simulink model "V2x_TC_TrafficLights_cs"  (Simulink 7.6)
settings: Solver=ode5  SolverMode=SingleTasking  StartTime=0.0  StopTime=38  FixedStep=1/100  RelTol=1e-3
PreLoadFcn:
  | check_NUTS_defined
  | 
  | assignin('base',['model_closed_' bdroot],false);addpath('../includes_V2x');
InitFcn:
  | PreScanExperimentInitFcn(bdroot)
StopFcn:
  | PreScanExperimentStopFcn(bdroot);
  | 
undefined symbols (not defined in the file or in its folder): acceleration, actorID, actorType, DiscIntNeverNeededParam, GPS_alt, GPS_lat, GPS_long, intmax, lightBarInUse, messageID, Newton, publicActorType, Raphson, rotZ, sigbuilder_block, sireneInUse, trafficLightPriority, turnAdvice, velocity, x_ini_VRML, y_ini_VRML, yawRate, z_ini_VRML
libraries: simulink x172, PreScanSimulinkLibrary x20, PreScanUsefulBlocks x11, simulink_extras x9, simulink_need_slupdate x1
(part 22 of 27)

== system V2x_TC_TrafficLights_cs/TrafficLightRoadSideNL_8/Infrastructure CAM generator/set CAM signals ==
  CAM generation signal : Inport
  warning : Inport  Port=2
  messageID : Inport  Port=3
  actorID : Inport  Port=4
  actorType : Inport  Port=5
  trafficLightPriority : Inport  Port=6
  GPS_lat : Inport  Port=7
  GPS_long : Inport  Port=8
  GPS_alt : Inport  Port=9
  rotZ : Inport  Port=10
  Mux : Mux  Inputs=9
  Switch : Switch  Criteria=u2 > Threshold  Threshold=0.5
  Unit Delay : UnitDelay  SampleTime=-1
  CAM_signals : Outport
  wires:
  rotZ/1 -> Mux/9
  GPS_alt/1 -> Mux/8
  GPS_long/1 -> Mux/7
  GPS_lat/1 -> Mux/6
  trafficLightPriority/1 -> Mux/5
  actorType/1 -> Mux/4
  actorID/1 -> Mux/3
  messageID/1 -> Mux/2
  warning/1 -> Mux/1
  Unit Delay/1 -> Switch/3
  Switch/1 -> CAM_signals/1, Unit Delay/1
  Mux/1 -> Switch/1
  CAM generation signal/1 -> Switch/2

== system V2x_TC_TrafficLights_cs/TrafficSignPoleNL_1/Infrastructure CAM generator/CAM generation ==
  Enable : EnablePort  StatesWhenEnabling=reset  PropagateVarSize=During execution
  Add : Sum  Inputs=+-
  Data Store Memory : DataStoreMemory  DataStoreName=CAM_time
  Data Store Read : DataStoreRead  DataStoreName=CAM_time  SampleTime=0.01
  Digital Clock : DigitalClock  SampleTime=0.01
  Merge : Merge
  if : If  IfExpression=u1 >= 1
  no : Subsystem  TreatAsAtomicUnit=on
  yes : Subsystem  TreatAsAtomicUnit=on
  CAN generation signal : Outport  OutputWhenDisabled=reset  InitialOutput=0
  wires:
  if/2 -> no/Action
  Merge/1 -> CAN generation signal/1
  no/1(Out1) -> Merge/2
  yes/1(Out1) -> Merge/1
  if/1 -> yes/Action
  Add/1 -> if/1
  Data Store Read/1 -> Add/2
  Digital Clock/1 -> Add/1

== system V2x_TC_TrafficLights_cs/TrafficSignPoleNL_1/Infrastructure CAM generator/Enabled Subsystem ==
  CAM_sig : Inport
  Enable : EnablePort  PropagateVarSize=During execution
  CAM_signals : Outport  OutputWhenDisabled=reset  InitialOutput=0
  wires:
  CAM_sig/1 -> CAM_signals/1

== system V2x_TC_TrafficLights_cs/TrafficSignPoleNL_1/Infrastructure CAM generator/coder ==
  R : Inport
  O : Inport  Port=2
  G : Inport  Port=3
  Add : Sum  Inputs=+++
  Gain : Gain  Gain=2
  Gain1 : Gain  Gain=3
  trafficLightPriority : Outport
  wires:
  Gain/1 -> Add/2
  Gain1/1 -> Add/3
  R/1 -> Add/1
  O/1 -> Gain/1
  Add/1 -> trafficLightPriority/1
  G/1 -> Gain1/1

== system V2x_TC_TrafficLights_cs/TrafficSignPoleNL_1/Infrastructure CAM generator/impulse generator ==
  CAM_time_step : Inport
  Add : Sum
  Compare To Zero : simulink/Logic and Bit Operations/Compare To Zero  SystemSampleTime=-1  relop=>  LogicOutDataTypeMode=boolean  ZeroCross=off
  Data Type Conversion : DataTypeConversion  OutDataTypeStr=boolean
  Detect Change : simulink/Logic and Bit Operations/Detect Change  SystemSampleTime=-1  vinit=0
  Digital Clock1 : DigitalClock  SampleTime=0.01
  Divide : Product  Inputs=*/
  Gain : Gain  Gain=pi
  Product : Product  RndMeth=Zero
  Trigonometric Function : Trigonometry
  constant : Constant
  experiment time step : Constant  Value=0.01  SampleTime=0
  enable : Outport
  wires:
  CAM_time_step/1 -> Divide/2
  Data Type Conversion/1 -> enable/1
  experiment time step/1 -> Add/2
  Add/1 -> Trigonometric Function/1
  Divide/1 -> Gain/1
  constant/1 -> Divide/1
  Product/1 -> Add/1
  Detect Change/1 -> Data Type Conversion/1
  Compare To Zero/1 -> Detect Change/1
  Trigonometric Function/1 -> Compare To Zero/1
  Gain/1 -> Product/2
  Digital Clock1/1 -> Product/1

== system V2x_TC_TrafficLights_cs/TrafficSignPoleNL_1/Infrastructure CAM generator/impulse generator1 ==
  CAM_time_step : Inport
  Add : Sum
  Compare To Zero : simulink/Logic and Bit Operations/Compare To Zero  SystemSampleTime=-1  relop=>  LogicOutDataTypeMode=boolean  ZeroCross=off
  Data Type Conversion : DataTypeConversion  OutDataTypeStr=boolean
  Detect Change : simulink/Logic and Bit Operations/Detect Change  SystemSampleTime=-1  vinit=0
  Digital Clock1 : DigitalClock  SampleTime=0.01
  Divide : Product  Inputs=*/
  Gain : Gain  Gain=pi
  Product : Product  RndMeth=Zero
  Trigonometric Function : Trigonometry
  constant : Constant
  experiment time step : Constant  Value=0.01  SampleTime=0
  enable : Outport
  wires:
  Digital Clock1/1 -> Product/1
  Gain/1 -> Product/2
  Trigonometric Function/1 -> Compare To Zero/1
  Compare To Zero/1 -> Detect Change/1
  Detect Change/1 -> Data Type Conversion/1
  Product/1 -> Add/1
  constant/1 -> Divide/1
  Divide/1 -> Gain/1
  Add/1 -> Trigonometric Function/1
  experiment time step/1 -> Add/2
  Data Type Conversion/1 -> enable/1
  CAM_time_step/1 -> Divide/2

== system V2x_TC_TrafficLights_cs/TrafficSignPoleNL_1/Infrastructure CAM generator/set CAM signals ==
  CAM generation signal : Inport
  warning : Inport  Port=2
  messageID : Inport  Port=3
  actorID : Inport  Port=4
  actorType : Inport  Port=5
  trafficLightPriority : Inport  Port=6
  GPS_lat : Inport  Port=7
  GPS_long : Inport  Port=8
  GPS_alt : Inport  Port=9
  rotZ : Inport  Port=10
  Mux : Mux  Inputs=9
  Switch : Switch  Criteria=u2 > Threshold  Threshold=0.5
  Unit Delay : UnitDelay  SampleTime=-1
  CAM_signals : Outport
  wires:
  rotZ/1 -> Mux/9
  GPS_alt/1 -> Mux/8
  GPS_long/1 -> Mux/7
  GPS_lat/1 -> Mux/6
  trafficLightPriority/1 -> Mux/5
  actorType/1 -> Mux/4
  actorID/1 -> Mux/3
  messageID/1 -> Mux/2
  warning/1 -> Mux/1
  Unit Delay/1 -> Switch/3
  Switch/1 -> CAM_signals/1, Unit Delay/1
  Mux/1 -> Switch/1
  CAM generation signal/1 -> Switch/2

== system V2x_TC_TrafficLights_cs/TrafficSignPoleNL_10/Infrastructure CAM generator/CAM generation ==
  Enable : EnablePort  StatesWhenEnabling=reset  PropagateVarSize=During execution
  Add : Sum  Inputs=+-
  Data Store Memory : DataStoreMemory  DataStoreName=CAM_time
  Data Store Read : DataStoreRead  DataStoreName=CAM_time  SampleTime=0.01
  Digital Clock : DigitalClock  SampleTime=0.01
  Merge : Merge
  if : If  IfExpression=u1 >= 1
  no : Subsystem  TreatAsAtomicUnit=on
  yes : Subsystem  TreatAsAtomicUnit=on
  CAN generation signal : Outport  OutputWhenDisabled=reset  InitialOutput=0
  wires:
  if/2 -> no/Action
  Merge/1 -> CAN generation signal/1
  no/1(Out1) -> Merge/2
  yes/1(Out1) -> Merge/1
  if/1 -> yes/Action
  Add/1 -> if/1
  Data Store Read/1 -> Add/2
  Digital Clock/1 -> Add/1

== system V2x_TC_TrafficLights_cs/TrafficSignPoleNL_10/Infrastructure CAM generator/Enabled Subsystem ==
  CAM_sig : Inport
  Enable : EnablePort  PropagateVarSize=During execution
  CAM_signals : Outport  OutputWhenDisabled=reset  InitialOutput=0
  wires:
  CAM_sig/1 -> CAM_signals/1

== system V2x_TC_TrafficLights_cs/TrafficSignPoleNL_10/Infrastructure CAM generator/coder ==
  R : Inport
  O : Inport  Port=2
  G : Inport  Port=3
  Add : Sum  Inputs=+++
  Gain : Gain  Gain=2
  Gain1 : Gain  Gain=3
  trafficLightPriority : Outport
  wires:
  Gain/1 -> Add/2
  Gain1/1 -> Add/3
  R/1 -> Add/1
  O/1 -> Gain/1
  Add/1 -> trafficLightPriority/1
  G/1 -> Gain1/1

== system V2x_TC_TrafficLights_cs/TrafficSignPoleNL_10/Infrastructure CAM generator/impulse generator ==
  CAM_time_step : Inport
  Add : Sum
  Compare To Zero : simulink/Logic and Bit Operations/Compare To Zero  SystemSampleTime=-1  relop=>  LogicOutDataTypeMode=boolean  ZeroCross=off
  Data Type Conversion : DataTypeConversion  OutDataTypeStr=boolean
  Detect Change : simulink/Logic and Bit Operations/Detect Change  SystemSampleTime=-1  vinit=0
  Digital Clock1 : DigitalClock  SampleTime=0.01
  Divide : Product  Inputs=*/
  Gain : Gain  Gain=pi
  Product : Product  RndMeth=Zero
  Trigonometric Function : Trigonometry
  constant : Constant
  experiment time step : Constant  Value=0.01  SampleTime=0
  enable : Outport
  wires:
  CAM_time_step/1 -> Divide/2
  Data Type Conversion/1 -> enable/1
  experiment time step/1 -> Add/2
  Add/1 -> Trigonometric Function/1
  Divide/1 -> Gain/1
  constant/1 -> Divide/1
  Product/1 -> Add/1
  Detect Change/1 -> Data Type Conversion/1
  Compare To Zero/1 -> Detect Change/1
  Trigonometric Function/1 -> Compare To Zero/1
  Gain/1 -> Product/2
  Digital Clock1/1 -> Product/1

== system V2x_TC_TrafficLights_cs/TrafficSignPoleNL_10/Infrastructure CAM generator/impulse generator1 ==
  CAM_time_step : Inport
  Add : Sum
  Compare To Zero : simulink/Logic and Bit Operations/Compare To Zero  SystemSampleTime=-1  relop=>  LogicOutDataTypeMode=boolean  ZeroCross=off
  Data Type Conversion : DataTypeConversion  OutDataTypeStr=boolean
  Detect Change : simulink/Logic and Bit Operations/Detect Change  SystemSampleTime=-1  vinit=0
  Digital Clock1 : DigitalClock  SampleTime=0.01
  Divide : Product  Inputs=*/
  Gain : Gain  Gain=pi
  Product : Product  RndMeth=Zero
  Trigonometric Function : Trigonometry
  constant : Constant
  experiment time step : Constant  Value=0.01  SampleTime=0
  enable : Outport
  wires:
  Digital Clock1/1 -> Product/1
  Gain/1 -> Product/2
  Trigonometric Function/1 -> Compare To Zero/1
  Compare To Zero/1 -> Detect Change/1
  Detect Change/1 -> Data Type Conversion/1
  Product/1 -> Add/1
  constant/1 -> Divide/1
  Divide/1 -> Gain/1
  Add/1 -> Trigonometric Function/1
  experiment time step/1 -> Add/2
  Data Type Conversion/1 -> enable/1
  CAM_time_step/1 -> Divide/2

== system V2x_TC_TrafficLights_cs/TrafficSignPoleNL_10/Infrastructure CAM generator/set CAM signals ==
  CAM generation signal : Inport
  warning : Inport  Port=2
  messageID : Inport  Port=3
  actorID : Inport  Port=4
  actorType : Inport  Port=5
  trafficLightPriority : Inport  Port=6
  GPS_lat : Inport  Port=7
  GPS_long : Inport  Port=8
  GPS_alt : Inport  Port=9
  rotZ : Inport  Port=10
  Mux : Mux  Inputs=9
  Switch : Switch  Criteria=u2 > Threshold  Threshold=0.5
  Unit Delay : UnitDelay  SampleTime=-1
  CAM_signals : Outport
  wires:
  rotZ/1 -> Mux/9
  GPS_alt/1 -> Mux/8
  GPS_long/1 -> Mux/7
  GPS_lat/1 -> Mux/6
  trafficLightPriority/1 -> Mux/5
  actorType/1 -> Mux/4
  actorID/1 -> Mux/3
  messageID/1 -> Mux/2
  warning/1 -> Mux/1
  Unit Delay/1 -> Switch/3
  Switch/1 -> CAM_signals/1, Unit Delay/1
  Mux/1 -> Switch/1
  CAM generation signal/1 -> Switch/2

== system V2x_TC_TrafficLights_cs/TrafficSignPoleNL_2/Infrastructure CAM generator/CAM generation ==
  Enable : EnablePort  StatesWhenEnabling=reset  PropagateVarSize=During execution
  Add : Sum  Inputs=+-
  Data Store Memory : DataStoreMemory  DataStoreName=CAM_time
  Data Store Read : DataStoreRead  DataStoreName=CAM_time  SampleTime=0.01
  Digital Clock : DigitalClock  SampleTime=0.01
  Merge : Merge
  if : If  IfExpression=u1 >= 1
  no : Subsystem  TreatAsAtomicUnit=on
  yes : Subsystem  TreatAsAtomicUnit=on
  CAN generation signal : Outport  OutputWhenDisabled=reset  InitialOutput=0
  wires:
  if/2 -> no/Action
  Merge/1 -> CAN generation signal/1
  no/1(Out1) -> Merge/2
  yes/1(Out1) -> Merge/1
  if/1 -> yes/Action
  Add/1 -> if/1
  Data Store Read/1 -> Add/2
  Digital Clock/1 -> Add/1

== system V2x_TC_TrafficLights_cs/TrafficSignPoleNL_2/Infrastructure CAM generator/Enabled Subsystem ==
  CAM_sig : Inport
  Enable : EnablePort  PropagateVarSize=During execution
  CAM_signals : Outport  OutputWhenDisabled=reset  InitialOutput=0
  wires:
  CAM_sig/1 -> CAM_signals/1

== system V2x_TC_TrafficLights_cs/TrafficSignPoleNL_2/Infrastructure CAM generator/coder ==
  R : Inport
  O : Inport  Port=2
  G : Inport  Port=3
  Add : Sum  Inputs=+++
  Gain : Gain  Gain=2
  Gain1 : Gain  Gain=3
  trafficLightPriority : Outport
  wires:
  Gain/1 -> Add/2
  Gain1/1 -> Add/3
  R/1 -> Add/1
  O/1 -> Gain/1
  Add/1 -> trafficLightPriority/1
  G/1 -> Gain1/1

== system V2x_TC_TrafficLights_cs/TrafficSignPoleNL_2/Infrastructure CAM generator/impulse generator ==
  CAM_time_step : Inport
  Add : Sum
  Compare To Zero : simulink/Logic and Bit Operations/Compare To Zero  SystemSampleTime=-1  relop=>  LogicOutDataTypeMode=boolean  ZeroCross=off
  Data Type Conversion : DataTypeConversion  OutDataTypeStr=boolean
  Detect Change : simulink/Logic and Bit Operations/Detect Change  SystemSampleTime=-1  vinit=0
  Digital Clock1 : DigitalClock  SampleTime=0.01
  Divide : Product  Inputs=*/
  Gain : Gain  Gain=pi
  Product : Product  RndMeth=Zero
  Trigonometric Function : Trigonometry
  constant : Constant
  experiment time step : Constant  Value=0.01  SampleTime=0
  enable : Outport
  wires:
  CAM_time_step/1 -> Divide/2
  Data Type Conversion/1 -> enable/1
  experiment time step/1 -> Add/2
  Add/1 -> Trigonometric Function/1
  Divide/1 -> Gain/1
  constant/1 -> Divide/1
  Product/1 -> Add/1
  Detect Change/1 -> Data Type Conversion/1
  Compare To Zero/1 -> Detect Change/1
  Trigonometric Function/1 -> Compare To Zero/1
  Gain/1 -> Product/2
  Digital Clock1/1 -> Product/1

== system V2x_TC_TrafficLights_cs/TrafficSignPoleNL_2/Infrastructure CAM generator/impulse generator1 ==
  CAM_time_step : Inport
  Add : Sum
  Compare To Zero : simulink/Logic and Bit Operations/Compare To Zero  SystemSampleTime=-1  relop=>  LogicOutDataTypeMode=boolean  ZeroCross=off
  Data Type Conversion : DataTypeConversion  OutDataTypeStr=boolean
  Detect Change : simulink/Logic and Bit Operations/Detect Change  SystemSampleTime=-1  vinit=0
  Digital Clock1 : DigitalClock  SampleTime=0.01
  Divide : Product  Inputs=*/
  Gain : Gain  Gain=pi
  Product : Product  RndMeth=Zero
  Trigonometric Function : Trigonometry
  constant : Constant
  experiment time step : Constant  Value=0.01  SampleTime=0
  enable : Outport
  wires:
  Digital Clock1/1 -> Product/1
  Gain/1 -> Product/2
  Trigonometric Function/1 -> Compare To Zero/1
  Compare To Zero/1 -> Detect Change/1
  Detect Change/1 -> Data Type Conversion/1
  Product/1 -> Add/1
  constant/1 -> Divide/1
  Divide/1 -> Gain/1
  Add/1 -> Trigonometric Function/1
  experiment time step/1 -> Add/2
  Data Type Conversion/1 -> enable/1
  CAM_time_step/1 -> Divide/2

== system V2x_TC_TrafficLights_cs/TrafficSignPoleNL_2/Infrastructure CAM generator/set CAM signals ==
  CAM generation signal : Inport
  warning : Inport  Port=2
  messageID : Inport  Port=3
  actorID : Inport  Port=4
  actorType : Inport  Port=5
  trafficLightPriority : Inport  Port=6
  GPS_lat : Inport  Port=7
  GPS_long : Inport  Port=8
  GPS_alt : Inport  Port=9
  rotZ : Inport  Port=10
  Mux : Mux  Inputs=9
  Switch : Switch  Criteria=u2 > Threshold  Threshold=0.5
  Unit Delay : UnitDelay  SampleTime=-1
  CAM_signals : Outport
  wires:
  rotZ/1 -> Mux/9
  GPS_alt/1 -> Mux/8
  GPS_long/1 -> Mux/7
  GPS_lat/1 -> Mux/6
  trafficLightPriority/1 -> Mux/5
  actorType/1 -> Mux/4
  actorID/1 -> Mux/3
  messageID/1 -> Mux/2
  warning/1 -> Mux/1
  Unit Delay/1 -> Switch/3
  Switch/1 -> CAM_signals/1, Unit Delay/1
  Mux/1 -> Switch/1
  CAM generation signal/1 -> Switch/2

== system V2x_TC_TrafficLights_cs/TrafficSignPoleNL_3/Infrastructure CAM generator/CAM generation ==
  Enable : EnablePort  StatesWhenEnabling=reset  PropagateVarSize=During execution
  Add : Sum  Inputs=+-
  Data Store Memory : DataStoreMemory  DataStoreName=CAM_time
  Data Store Read : DataStoreRead  DataStoreName=CAM_time  SampleTime=0.01
  Digital Clock : DigitalClock  SampleTime=0.01
  Merge : Merge
  if : If  IfExpression=u1 >= 1
  no : Subsystem  TreatAsAtomicUnit=on
  yes : Subsystem  TreatAsAtomicUnit=on
  CAN generation signal : Outport  OutputWhenDisabled=reset  InitialOutput=0
  wires:
  if/2 -> no/Action
  Merge/1 -> CAN generation signal/1
  no/1(Out1) -> Merge/2
  yes/1(Out1) -> Merge/1
  if/1 -> yes/Action
  Add/1 -> if/1
  Data Store Read/1 -> Add/2
  Digital Clock/1 -> Add/1

== system V2x_TC_TrafficLights_cs/TrafficSignPoleNL_3/Infrastructure CAM generator/Enabled Subsystem ==
  CAM_sig : Inport
  Enable : EnablePort  PropagateVarSize=During execution
  CAM_signals : Outport  OutputWhenDisabled=reset  InitialOutput=0
  wires:
  CAM_sig/1 -> CAM_signals/1

== system V2x_TC_TrafficLights_cs/TrafficSignPoleNL_3/Infrastructure CAM generator/coder ==
  R : Inport
  O : Inport  Port=2
  G : Inport  Port=3
  Add : Sum  Inputs=+++
  Gain : Gain  Gain=2
  Gain1 : Gain  Gain=3
  trafficLightPriority : Outport
  wires:
  Gain/1 -> Add/2
  Gain1/1 -> Add/3
  R/1 -> Add/1
  O/1 -> Gain/1
  Add/1 -> trafficLightPriority/1
  G/1 -> Gain1/1

== system V2x_TC_TrafficLights_cs/TrafficSignPoleNL_3/Infrastructure CAM generator/impulse generator ==
  CAM_time_step : Inport
  Add : Sum
  Compare To Zero : simulink/Logic and Bit Operations/Compare To Zero  SystemSampleTime=-1  relop=>  LogicOutDataTypeMode=boolean  ZeroCross=off
  Data Type Conversion : DataTypeConversion  OutDataTypeStr=boolean
  Detect Change : simulink/Logic and Bit Operations/Detect Change  SystemSampleTime=-1  vinit=0
  Digital Clock1 : DigitalClock  SampleTime=0.01
  Divide : Product  Inputs=*/
  Gain : Gain  Gain=pi
  Product : Product  RndMeth=Zero
  Trigonometric Function : Trigonometry
  constant : Constant
  experiment time step : Constant  Value=0.01  SampleTime=0
  enable : Outport
  wires:
  CAM_time_step/1 -> Divide/2
  Data Type Conversion/1 -> enable/1
  experiment time step/1 -> Add/2
  Add/1 -> Trigonometric Function/1
  Divide/1 -> Gain/1
  constant/1 -> Divide/1
  Product/1 -> Add/1
  Detect Change/1 -> Data Type Conversion/1
  Compare To Zero/1 -> Detect Change/1
  Trigonometric Function/1 -> Compare To Zero/1
  Gain/1 -> Product/2
  Digital Clock1/1 -> Product/1

== system V2x_TC_TrafficLights_cs/TrafficSignPoleNL_3/Infrastructure CAM generator/impulse generator1 ==
  CAM_time_step : Inport
  Add : Sum
  Compare To Zero : simulink/Logic and Bit Operations/Compare To Zero  SystemSampleTime=-1  relop=>  LogicOutDataTypeMode=boolean  ZeroCross=off
  Data Type Conversion : DataTypeConversion  OutDataTypeStr=boolean
  Detect Change : simulink/Logic and Bit Operations/Detect Change  SystemSampleTime=-1  vinit=0
  Digital Clock1 : DigitalClock  SampleTime=0.01
  Divide : Product  Inputs=*/
  Gain : Gain  Gain=pi
  Product : Product  RndMeth=Zero
  Trigonometric Function : Trigonometry
  constant : Constant
  experiment time step : Constant  Value=0.01  SampleTime=0
  enable : Outport
  wires:
  Digital Clock1/1 -> Product/1
  Gain/1 -> Product/2
  Trigonometric Function/1 -> Compare To Zero/1
  Compare To Zero/1 -> Detect Change/1
  Detect Change/1 -> Data Type Conversion/1
  Product/1 -> Add/1
  constant/1 -> Divide/1
  Divide/1 -> Gain/1
  Add/1 -> Trigonometric Function/1
  experiment time step/1 -> Add/2
  Data Type Conversion/1 -> enable/1
  CAM_time_step/1 -> Divide/2

== system V2x_TC_TrafficLights_cs/TrafficSignPoleNL_3/Infrastructure CAM generator/set CAM signals ==
  CAM generation signal : Inport
  warning : Inport  Port=2
  messageID : Inport  Port=3
  actorID : Inport  Port=4
  actorType : Inport  Port=5
  trafficLightPriority : Inport  Port=6
  GPS_lat : Inport  Port=7
  GPS_long : Inport  Port=8
  GPS_alt : Inport  Port=9
  rotZ : Inport  Port=10
  Mux : Mux  Inputs=9
  Switch : Switch  Criteria=u2 > Threshold  Threshold=0.5
  Unit Delay : UnitDelay  SampleTime=-1
  CAM_signals : Outport
  wires:
  rotZ/1 -> Mux/9
  GPS_alt/1 -> Mux/8
  GPS_long/1 -> Mux/7
  GPS_lat/1 -> Mux/6
  trafficLightPriority/1 -> Mux/5
  actorType/1 -> Mux/4
  actorID/1 -> Mux/3
  messageID/1 -> Mux/2
  warning/1 -> Mux/1
  Unit Delay/1 -> Switch/3
  Switch/1 -> CAM_signals/1, Unit Delay/1
  Mux/1 -> Switch/1
  CAM generation signal/1 -> Switch/2

== system V2x_TC_TrafficLights_cs/TrafficSignPoleNL_4/Infrastructure CAM generator/CAM generation ==
  Enable : EnablePort  StatesWhenEnabling=reset  PropagateVarSize=During execution
  Add : Sum  Inputs=+-
  Data Store Memory : DataStoreMemory  DataStoreName=CAM_time
  Data Store Read : DataStoreRead  DataStoreName=CAM_time  SampleTime=0.01
  Digital Clock : DigitalClock  SampleTime=0.01
  Merge : Merge
  if : If  IfExpression=u1 >= 1
  no : Subsystem  TreatAsAtomicUnit=on
  yes : Subsystem  TreatAsAtomicUnit=on
  CAN generation signal : Outport  OutputWhenDisabled=reset  InitialOutput=0
  wires:
  if/2 -> no/Action
  Merge/1 -> CAN generation signal/1
  no/1(Out1) -> Merge/2
  yes/1(Out1) -> Merge/1
  if/1 -> yes/Action
  Add/1 -> if/1
  Data Store Read/1 -> Add/2
  Digital Clock/1 -> Add/1

== system V2x_TC_TrafficLights_cs/TrafficSignPoleNL_4/Infrastructure CAM generator/Enabled Subsystem ==
  CAM_sig : Inport
  Enable : EnablePort  PropagateVarSize=During execution
  CAM_signals : Outport  OutputWhenDisabled=reset  InitialOutput=0
  wires:
  CAM_sig/1 -> CAM_signals/1

== system V2x_TC_TrafficLights_cs/TrafficSignPoleNL_4/Infrastructure CAM generator/coder ==
  R : Inport
  O : Inport  Port=2
  G : Inport  Port=3
  Add : Sum  Inputs=+++
  Gain : Gain  Gain=2
  Gain1 : Gain  Gain=3
  trafficLightPriority : Outport
  wires:
  Gain/1 -> Add/2
  Gain1/1 -> Add/3
  R/1 -> Add/1
  O/1 -> Gain/1
  Add/1 -> trafficLightPriority/1
  G/1 -> Gain1/1

== system V2x_TC_TrafficLights_cs/TrafficSignPoleNL_4/Infrastructure CAM generator/impulse generator ==
  CAM_time_step : Inport
  Add : Sum
  Compare To Zero : simulink/Logic and Bit Operations/Compare To Zero  SystemSampleTime=-1  relop=>  LogicOutDataTypeMode=boolean  ZeroCross=off
  Data Type Conversion : DataTypeConversion  OutDataTypeStr=boolean
  Detect Change : simulink/Logic and Bit Operations/Detect Change  SystemSampleTime=-1  vinit=0
  Digital Clock1 : DigitalClock  SampleTime=0.01
  Divide : Product  Inputs=*/
  Gain : Gain  Gain=pi
  Product : Product  RndMeth=Zero
  Trigonometric Function : Trigonometry
  constant : Constant
  experiment time step : Constant  Value=0.01  SampleTime=0
  enable : Outport
  wires:
  CAM_time_step/1 -> Divide/2
  Data Type Conversion/1 -> enable/1
  experiment time step/1 -> Add/2
  Add/1 -> Trigonometric Function/1
  Divide/1 -> Gain/1
  constant/1 -> Divide/1
  Product/1 -> Add/1
  Detect Change/1 -> Data Type Conversion/1
  Compare To Zero/1 -> Detect Change/1
  Trigonometric Function/1 -> Compare To Zero/1
  Gain/1 -> Product/2
  Digital Clock1/1 -> Product/1

== system V2x_TC_TrafficLights_cs/TrafficSignPoleNL_4/Infrastructure CAM generator/impulse generator1 ==
  CAM_time_step : Inport
  Add : Sum
  Compare To Zero : simulink/Logic and Bit Operations/Compare To Zero  SystemSampleTime=-1  relop=>  LogicOutDataTypeMode=boolean  ZeroCross=off
  Data Type Conversion : DataTypeConversion  OutDataTypeStr=boolean
  Detect Change : simulink/Logic and Bit Operations/Detect Change  SystemSampleTime=-1  vinit=0
  Digital Clock1 : DigitalClock  SampleTime=0.01
  Divide : Product  Inputs=*/
  Gain : Gain  Gain=pi
  Product : Product  RndMeth=Zero
  Trigonometric Function : Trigonometry
  constant : Constant
  experiment time step : Constant  Value=0.01  SampleTime=0
  enable : Outport
  wires:
  Digital Clock1/1 -> Product/1
  Gain/1 -> Product/2
  Trigonometric Function/1 -> Compare To Zero/1
  Compare To Zero/1 -> Detect Change/1
  Detect Change/1 -> Data Type Conversion/1
  Product/1 -> Add/1
  constant/1 -> Divide/1
  Divide/1 -> Gain/1
  Add/1 -> Trigonometric Function/1
  experiment time step/1 -> Add/2
  Data Type Conversion/1 -> enable/1
  CAM_time_step/1 -> Divide/2

== system V2x_TC_TrafficLights_cs/TrafficSignPoleNL_4/Infrastructure CAM generator/set CAM signals ==
  CAM generation signal : Inport
  warning : Inport  Port=2
  messageID : Inport  Port=3
  actorID : Inport  Port=4
  actorType : Inport  Port=5
  trafficLightPriority : Inport  Port=6
  GPS_lat : Inport  Port=7
  GPS_long : Inport  Port=8
  GPS_alt : Inport  Port=9
  rotZ : Inport  Port=10
  Mux : Mux  Inputs=9
  Switch : Switch  Criteria=u2 > Threshold  Threshold=0.5
  Unit Delay : UnitDelay  SampleTime=-1
  CAM_signals : Outport
  wires:
  rotZ/1 -> Mux/9
  GPS_alt/1 -> Mux/8
  GPS_long/1 -> Mux/7
  GPS_lat/1 -> Mux/6
  trafficLightPriority/1 -> Mux/5
  actorType/1 -> Mux/4
  actorID/1 -> Mux/3
  messageID/1 -> Mux/2
  warning/1 -> Mux/1
  Unit Delay/1 -> Switch/3
  Switch/1 -> CAM_signals/1, Unit Delay/1
  Mux/1 -> Switch/1
  CAM generation signal/1 -> Switch/2

== system V2x_TC_TrafficLights_cs/TrafficSignPoleNL_5/Infrastructure CAM generator/CAM generation ==
  Enable : EnablePort  StatesWhenEnabling=reset  PropagateVarSize=During execution
  Add : Sum  Inputs=+-
  Data Store Memory : DataStoreMemory  DataStoreName=CAM_time
  Data Store Read : DataStoreRead  DataStoreName=CAM_time  SampleTime=0.01
  Digital Clock : DigitalClock  SampleTime=0.01
  Merge : Merge
  if : If  IfExpression=u1 >= 1
  no : Subsystem  TreatAsAtomicUnit=on
  yes : Subsystem  TreatAsAtomicUnit=on
  CAN generation signal : Outport  OutputWhenDisabled=reset  InitialOutput=0
  wires:
  if/2 -> no/Action
  Merge/1 -> CAN generation signal/1
  no/1(Out1) -> Merge/2
  yes/1(Out1) -> Merge/1
  if/1 -> yes/Action
  Add/1 -> if/1
  Data Store Read/1 -> Add/2
  Digital Clock/1 -> Add/1

== system V2x_TC_TrafficLights_cs/TrafficSignPoleNL_5/Infrastructure CAM generator/Enabled Subsystem ==
  CAM_sig : Inport
  Enable : EnablePort  PropagateVarSize=During execution
  CAM_signals : Outport  OutputWhenDisabled=reset  InitialOutput=0
  wires:
  CAM_sig/1 -> CAM_signals/1

== system V2x_TC_TrafficLights_cs/TrafficSignPoleNL_5/Infrastructure CAM generator/coder ==
  R : Inport
  O : Inport  Port=2
  G : Inport  Port=3
  Add : Sum  Inputs=+++
  Gain : Gain  Gain=2
  Gain1 : Gain  Gain=3
  trafficLightPriority : Outport
  wires:
  Gain/1 -> Add/2
  Gain1/1 -> Add/3
  R/1 -> Add/1
  O/1 -> Gain/1
  Add/1 -> trafficLightPriority/1
  G/1 -> Gain1/1

== system V2x_TC_TrafficLights_cs/TrafficSignPoleNL_5/Infrastructure CAM generator/impulse generator ==
  CAM_time_step : Inport
  Add : Sum
  Compare To Zero : simulink/Logic and Bit Operations/Compare To Zero  SystemSampleTime=-1  relop=>  LogicOutDataTypeMode=boolean  ZeroCross=off
  Data Type Conversion : DataTypeConversion  OutDataTypeStr=boolean
  Detect Change : simulink/Logic and Bit Operations/Detect Change  SystemSampleTime=-1  vinit=0
  Digital Clock1 : DigitalClock  SampleTime=0.01
  Divide : Product  Inputs=*/
  Gain : Gain  Gain=pi
  Product : Product  RndMeth=Zero
  Trigonometric Function : Trigonometry
  constant : Constant
  experiment time step : Constant  Value=0.01  SampleTime=0
  enable : Outport
  wires:
  CAM_time_step/1 -> Divide/2
  Data Type Conversion/1 -> enable/1
  experiment time step/1 -> Add/2
  Add/1 -> Trigonometric Function/1
  Divide/1 -> Gain/1
  constant/1 -> Divide/1
  Product/1 -> Add/1
  Detect Change/1 -> Data Type Conversion/1
  Compare To Zero/1 -> Detect Change/1
  Trigonometric Function/1 -> Compare To Zero/1
  Gain/1 -> Product/2
  Digital Clock1/1 -> Product/1

== system V2x_TC_TrafficLights_cs/TrafficSignPoleNL_5/Infrastructure CAM generator/impulse generator1 ==
  CAM_time_step : Inport
  Add : Sum
  Compare To Zero : simulink/Logic and Bit Operations/Compare To Zero  SystemSampleTime=-1  relop=>  LogicOutDataTypeMode=boolean  ZeroCross=off
  Data Type Conversion : DataTypeConversion  OutDataTypeStr=boolean
  Detect Change : simulink/Logic and Bit Operations/Detect Change  SystemSampleTime=-1  vinit=0
  Digital Clock1 : DigitalClock  SampleTime=0.01
  Divide : Product  Inputs=*/
  Gain : Gain  Gain=pi
  Product : Product  RndMeth=Zero
  Trigonometric Function : Trigonometry
  constant : Constant
  experiment time step : Constant  Value=0.01  SampleTime=0
  enable : Outport
  wires:
  Digital Clock1/1 -> Product/1
  Gain/1 -> Product/2
  Trigonometric Function/1 -> Compare To Zero/1
  Compare To Zero/1 -> Detect Change/1
  Detect Change/1 -> Data Type Conversion/1
  Product/1 -> Add/1
  constant/1 -> Divide/1
  Divide/1 -> Gain/1
  Add/1 -> Trigonometric Function/1
  experiment time step/1 -> Add/2
  Data Type Conversion/1 -> enable/1
  CAM_time_step/1 -> Divide/2

== system V2x_TC_TrafficLights_cs/TrafficSignPoleNL_5/Infrastructure CAM generator/set CAM signals ==
  CAM generation signal : Inport
  warning : Inport  Port=2
  messageID : Inport  Port=3
  actorID : Inport  Port=4
  actorType : Inport  Port=5
  trafficLightPriority : Inport  Port=6
  GPS_lat : Inport  Port=7
  GPS_long : Inport  Port=8
  GPS_alt : Inport  Port=9
  rotZ : Inport  Port=10
  Mux : Mux  Inputs=9
  Switch : Switch  Criteria=u2 > Threshold  Threshold=0.5
  Unit Delay : UnitDelay  SampleTime=-1
  CAM_signals : Outport
  wires:
  rotZ/1 -> Mux/9
  GPS_alt/1 -> Mux/8
  GPS_long/1 -> Mux/7
  GPS_lat/1 -> Mux/6
  trafficLightPriority/1 -> Mux/5
  actorType/1 -> Mux/4
  actorID/1 -> Mux/3
  messageID/1 -> Mux/2
  warning/1 -> Mux/1
  Unit Delay/1 -> Switch/3
  Switch/1 -> CAM_signals/1, Unit Delay/1
  Mux/1 -> Switch/1
  CAM generation signal/1 -> Switch/2

== system V2x_TC_TrafficLights_cs/TrafficSignPoleNL_6/Infrastructure CAM generator/CAM generation ==
  Enable : EnablePort  StatesWhenEnabling=reset  PropagateVarSize=During execution
  Add : Sum  Inputs=+-
  Data Store Memory : DataStoreMemory  DataStoreName=CAM_time
  Data Store Read : DataStoreRead  DataStoreName=CAM_time  SampleTime=0.01
  Digital Clock : DigitalClock  SampleTime=0.01
  Merge : Merge
  if : If  IfExpression=u1 >= 1
  no : Subsystem  TreatAsAtomicUnit=on
  yes : Subsystem  TreatAsAtomicUnit=on
  CAN generation signal : Outport  OutputWhenDisabled=reset  InitialOutput=0
  wires:
  if/2 -> no/Action
  Merge/1 -> CAN generation signal/1
  no/1(Out1) -> Merge/2
  yes/1(Out1) -> Merge/1
  if/1 -> yes/Action
  Add/1 -> if/1
  Data Store Read/1 -> Add/2
  Digital Clock/1 -> Add/1

== system V2x_TC_TrafficLights_cs/TrafficSignPoleNL_6/Infrastructure CAM generator/Enabled Subsystem ==
  CAM_sig : Inport
  Enable : EnablePort  PropagateVarSize=During execution
  CAM_signals : Outport  OutputWhenDisabled=reset  InitialOutput=0
  wires:
  CAM_sig/1 -> CAM_signals/1

== system V2x_TC_TrafficLights_cs/TrafficSignPoleNL_6/Infrastructure CAM generator/coder ==
  R : Inport
  O : Inport  Port=2
  G : Inport  Port=3
  Add : Sum  Inputs=+++
  Gain : Gain  Gain=2
  Gain1 : Gain  Gain=3
  trafficLightPriority : Outport
  wires:
  Gain/1 -> Add/2
  Gain1/1 -> Add/3
  R/1 -> Add/1
  O/1 -> Gain/1
  Add/1 -> trafficLightPriority/1
  G/1 -> Gain1/1

== system V2x_TC_TrafficLights_cs/TrafficSignPoleNL_6/Infrastructure CAM generator/impulse generator ==
  CAM_time_step : Inport
  Add : Sum
  Compare To Zero : simulink/Logic and Bit Operations/Compare To Zero  SystemSampleTime=-1  relop=>  LogicOutDataTypeMode=boolean  ZeroCross=off
  Data Type Conversion : DataTypeConversion  OutDataTypeStr=boolean
  Detect Change : simulink/Logic and Bit Operations/Detect Change  SystemSampleTime=-1  vinit=0
  Digital Clock1 : DigitalClock  SampleTime=0.01
  Divide : Product  Inputs=*/
  Gain : Gain  Gain=pi
  Product : Product  RndMeth=Zero
  Trigonometric Function : Trigonometry
  constant : Constant
  experiment time step : Constant  Value=0.01  SampleTime=0
  enable : Outport
  wires:
  CAM_time_step/1 -> Divide/2
  Data Type Conversion/1 -> enable/1
  experiment time step/1 -> Add/2
  Add/1 -> Trigonometric Function/1
  Divide/1 -> Gain/1
  constant/1 -> Divide/1
  Product/1 -> Add/1
  Detect Change/1 -> Data Type Conversion/1
  Compare To Zero/1 -> Detect Change/1
  Trigonometric Function/1 -> Compare To Zero/1
  Gain/1 -> Product/2
  Digital Clock1/1 -> Product/1

== system V2x_TC_TrafficLights_cs/TrafficSignPoleNL_6/Infrastructure CAM generator/impulse generator1 ==
  CAM_time_step : Inport
  Add : Sum
  Compare To Zero : simulink/Logic and Bit Operations/Compare To Zero  SystemSampleTime=-1  relop=>  LogicOutDataTypeMode=boolean  ZeroCross=off
  Data Type Conversion : DataTypeConversion  OutDataTypeStr=boolean
  Detect Change : simulink/Logic and Bit Operations/Detect Change  SystemSampleTime=-1  vinit=0
  Digital Clock1 : DigitalClock  SampleTime=0.01
  Divide : Product  Inputs=*/
  Gain : Gain  Gain=pi
  Product : Product  RndMeth=Zero
  Trigonometric Function : Trigonometry
  constant : Constant
  experiment time step : Constant  Value=0.01  SampleTime=0
  enable : Outport
  wires:
  Digital Clock1/1 -> Product/1
  Gain/1 -> Product/2
  Trigonometric Function/1 -> Compare To Zero/1
  Compare To Zero/1 -> Detect Change/1
  Detect Change/1 -> Data Type Conversion/1
  Product/1 -> Add/1
  constant/1 -> Divide/1
  Divide/1 -> Gain/1
  Add/1 -> Trigonometric Function/1
  experiment time step/1 -> Add/2
  Data Type Conversion/1 -> enable/1
  CAM_time_step/1 -> Divide/2

== system V2x_TC_TrafficLights_cs/TrafficSignPoleNL_6/Infrastructure CAM generator/set CAM signals ==
  CAM generation signal : Inport
  warning : Inport  Port=2
  messageID : Inport  Port=3
  actorID : Inport  Port=4
  actorType : Inport  Port=5
  trafficLightPriority : Inport  Port=6
  GPS_lat : Inport  Port=7
  GPS_long : Inport  Port=8
  GPS_alt : Inport  Port=9
  rotZ : Inport  Port=10
  Mux : Mux  Inputs=9
  Switch : Switch  Criteria=u2 > Threshold  Threshold=0.5
  Unit Delay : UnitDelay  SampleTime=-1
  CAM_signals : Outport
  wires:
  rotZ/1 -> Mux/9
  GPS_alt/1 -> Mux/8
  GPS_long/1 -> Mux/7
  GPS_lat/1 -> Mux/6
  trafficLightPriority/1 -> Mux/5
  actorType/1 -> Mux/4
  actorID/1 -> Mux/3
  messageID/1 -> Mux/2
  warning/1 -> Mux/1
  Unit Delay/1 -> Switch/3
  Switch/1 -> CAM_signals/1, Unit Delay/1
  Mux/1 -> Switch/1
  CAM generation signal/1 -> Switch/2

== system V2x_TC_TrafficLights_cs/TrafficSignPoleNL_7/Infrastructure CAM generator/CAM generation ==
  Enable : EnablePort  StatesWhenEnabling=reset  PropagateVarSize=During execution
  Add : Sum  Inputs=+-
  Data Store Memory : DataStoreMemory  DataStoreName=CAM_time
  Data Store Read : DataStoreRead  DataStoreName=CAM_time  SampleTime=0.01
  Digital Clock : DigitalClock  SampleTime=0.01
  Merge : Merge
  if : If  IfExpression=u1 >= 1
  no : Subsystem  TreatAsAtomicUnit=on
  yes : Subsystem  TreatAsAtomicUnit=on
  CAN generation signal : Outport  OutputWhenDisabled=reset  InitialOutput=0
  wires:
  if/2 -> no/Action
  Merge/1 -> CAN generation signal/1
  no/1(Out1) -> Merge/2
  yes/1(Out1) -> Merge/1
  if/1 -> yes/Action
  Add/1 -> if/1
  Data Store Read/1 -> Add/2
  Digital Clock/1 -> Add/1

== system V2x_TC_TrafficLights_cs/TrafficSignPoleNL_7/Infrastructure CAM generator/Enabled Subsystem ==
  CAM_sig : Inport
  Enable : EnablePort  PropagateVarSize=During execution
  CAM_signals : Outport  OutputWhenDisabled=reset  InitialOutput=0
  wires:
  CAM_sig/1 -> CAM_signals/1

== system V2x_TC_TrafficLights_cs/TrafficSignPoleNL_7/Infrastructure CAM generator/coder ==
  R : Inport
  O : Inport  Port=2
  G : Inport  Port=3
  Add : Sum  Inputs=+++
  Gain : Gain  Gain=2
  Gain1 : Gain  Gain=3
  trafficLightPriority : Outport
  wires:
  Gain/1 -> Add/2
  Gain1/1 -> Add/3
  R/1 -> Add/1
  O/1 -> Gain/1
  Add/1 -> trafficLightPriority/1
  G/1 -> Gain1/1

== system V2x_TC_TrafficLights_cs/TrafficSignPoleNL_7/Infrastructure CAM generator/impulse generator ==
  CAM_time_step : Inport
  Add : Sum
  Compare To Zero : simulink/Logic and Bit Operations/Compare To Zero  SystemSampleTime=-1  relop=>  LogicOutDataTypeMode=boolean  ZeroCross=off
  Data Type Conversion : DataTypeConversion  OutDataTypeStr=boolean
  Detect Change : simulink/Logic and Bit Operations/Detect Change  SystemSampleTime=-1  vinit=0
  Digital Clock1 : DigitalClock  SampleTime=0.01
  Divide : Product  Inputs=*/
  Gain : Gain  Gain=pi
  Product : Product  RndMeth=Zero
  Trigonometric Function : Trigonometry
  constant : Constant
  experiment time step : Constant  Value=0.01  SampleTime=0
  enable : Outport
  wires:
  CAM_time_step/1 -> Divide/2
  Data Type Conversion/1 -> enable/1
  experiment time step/1 -> Add/2
  Add/1 -> Trigonometric Function/1
  Divide/1 -> Gain/1
  constant/1 -> Divide/1
  Product/1 -> Add/1
  Detect Change/1 -> Data Type Conversion/1
  Compare To Zero/1 -> Detect Change/1
  Trigonometric Function/1 -> Compare To Zero/1
  Gain/1 -> Product/2
  Digital Clock1/1 -> Product/1

== system V2x_TC_TrafficLights_cs/TrafficSignPoleNL_7/Infrastructure CAM generator/impulse generator1 ==
  CAM_time_step : Inport
  Add : Sum
  Compare To Zero : simulink/Logic and Bit Operations/Compare To Zero  SystemSampleTime=-1  relop=>  LogicOutDataTypeMode=boolean  ZeroCross=off
  Data Type Conversion : DataTypeConversion  OutDataTypeStr=boolean
  Detect Change : simulink/Logic and Bit Operations/Detect Change  SystemSampleTime=-1  vinit=0
  Digital Clock1 : DigitalClock  SampleTime=0.01
  Divide : Product  Inputs=*/
  Gain : Gain  Gain=pi
  Product : Product  RndMeth=Zero
  Trigonometric Function : Trigonometry
  constant : Constant
  experiment time step : Constant  Value=0.01  SampleTime=0
  enable : Outport
  wires:
  Digital Clock1/1 -> Product/1
  Gain/1 -> Product/2
  Trigonometric Function/1 -> Compare To Zero/1
  Compare To Zero/1 -> Detect Change/1
  Detect Change/1 -> Data Type Conversion/1
  Product/1 -> Add/1
  constant/1 -> Divide/1
  Divide/1 -> Gain/1
  Add/1 -> Trigonometric Function/1
  experiment time step/1 -> Add/2
  Data Type Conversion/1 -> enable/1
  CAM_time_step/1 -> Divide/2

== system V2x_TC_TrafficLights_cs/TrafficSignPoleNL_7/Infrastructure CAM generator/set CAM signals ==
  CAM generation signal : Inport
  warning : Inport  Port=2
  messageID : Inport  Port=3
  actorID : Inport  Port=4
  actorType : Inport  Port=5
  trafficLightPriority : Inport  Port=6
  GPS_lat : Inport  Port=7
  GPS_long : Inport  Port=8
  GPS_alt : Inport  Port=9
  rotZ : Inport  Port=10
  Mux : Mux  Inputs=9
  Switch : Switch  Criteria=u2 > Threshold  Threshold=0.5
  Unit Delay : UnitDelay  SampleTime=-1
  CAM_signals : Outport
  wires:
  rotZ/1 -> Mux/9
  GPS_alt/1 -> Mux/8
  GPS_long/1 -> Mux/7
  GPS_lat/1 -> Mux/6
  trafficLightPriority/1 -> Mux/5
  actorType/1 -> Mux/4
  actorID/1 -> Mux/3
  messageID/1 -> Mux/2
  warning/1 -> Mux/1
  Unit Delay/1 -> Switch/3
  Switch/1 -> CAM_signals/1, Unit Delay/1
  Mux/1 -> Switch/1
  CAM generation signal/1 -> Switch/2

== system V2x_TC_TrafficLights_cs/TrafficSignPoleNL_8/Infrastructure CAM generator/CAM generation ==
  Enable : EnablePort  StatesWhenEnabling=reset  PropagateVarSize=During execution
  Add : Sum  Inputs=+-
  Data Store Memory : DataStoreMemory  DataStoreName=CAM_time
  Data Store Read : DataStoreRead  DataStoreName=CAM_time  SampleTime=0.01
  Digital Clock : DigitalClock  SampleTime=0.01
  Merge : Merge
  if : If  IfExpression=u1 >= 1
  no : Subsystem  TreatAsAtomicUnit=on
  yes : Subsystem  TreatAsAtomicUnit=on
  CAN generation signal : Outport  OutputWhenDisabled=reset  InitialOutput=0
  wires:
  if/2 -> no/Action
  Merge/1 -> CAN generation signal/1
  no/1(Out1) -> Merge/2
  yes/1(Out1) -> Merge/1
  if/1 -> yes/Action
  Add/1 -> if/1
  Data Store Read/1 -> Add/2
  Digital Clock/1 -> Add/1

== system V2x_TC_TrafficLights_cs/TrafficSignPoleNL_8/Infrastructure CAM generator/Enabled Subsystem ==
  CAM_sig : Inport
  Enable : EnablePort  PropagateVarSize=During execution
  CAM_signals : Outport  OutputWhenDisabled=reset  InitialOutput=0
  wires:
  CAM_sig/1 -> CAM_signals/1

== system V2x_TC_TrafficLights_cs/TrafficSignPoleNL_8/Infrastructure CAM generator/coder ==
  R : Inport
  O : Inport  Port=2
  G : Inport  Port=3
  Add : Sum  Inputs=+++
  Gain : Gain  Gain=2
  Gain1 : Gain  Gain=3
  trafficLightPriority : Outport
  wires:
  Gain/1 -> Add/2
  Gain1/1 -> Add/3
  R/1 -> Add/1
  O/1 -> Gain/1
  Add/1 -> trafficLightPriority/1
  G/1 -> Gain1/1

== system V2x_TC_TrafficLights_cs/TrafficSignPoleNL_8/Infrastructure CAM generator/impulse generator ==
  CAM_time_step : Inport
  Add : Sum
  Compare To Zero : simulink/Logic and Bit Operations/Compare To Zero  SystemSampleTime=-1  relop=>  LogicOutDataTypeMode=boolean  ZeroCross=off
  Data Type Conversion : DataTypeConversion  OutDataTypeStr=boolean
  Detect Change : simulink/Logic and Bit Operations/Detect Change  SystemSampleTime=-1  vinit=0
  Digital Clock1 : DigitalClock  SampleTime=0.01
  Divide : Product  Inputs=*/
  Gain : Gain  Gain=pi
  Product : Product  RndMeth=Zero
  Trigonometric Function : Trigonometry
  constant : Constant
  experiment time step : Constant  Value=0.01  SampleTime=0
  enable : Outport
  wires:
  CAM_time_step/1 -> Divide/2
  Data Type Conversion/1 -> enable/1
  experiment time step/1 -> Add/2
  Add/1 -> Trigonometric Function/1
  Divide/1 -> Gain/1
  constant/1 -> Divide/1
  Product/1 -> Add/1
  Detect Change/1 -> Data Type Conversion/1
  Compare To Zero/1 -> Detect Change/1
  Trigonometric Function/1 -> Compare To Zero/1
  Gain/1 -> Product/2
  Digital Clock1/1 -> Product/1

== system V2x_TC_TrafficLights_cs/TrafficSignPoleNL_8/Infrastructure CAM generator/impulse generator1 ==
  CAM_time_step : Inport
  Add : Sum
  Compare To Zero : simulink/Logic and Bit Operations/Compare To Zero  SystemSampleTime=-1  relop=>  LogicOutDataTypeMode=boolean  ZeroCross=off
  Data Type Conversion : DataTypeConversion  OutDataTypeStr=boolean
  Detect Change : simulink/Logic and Bit Operations/Detect Change  SystemSampleTime=-1  vinit=0
  Digital Clock1 : DigitalClock  SampleTime=0.01
  Divide : Product  Inputs=*/
  Gain : Gain  Gain=pi
  Product : Product  RndMeth=Zero
  Trigonometric Function : Trigonometry
  constant : Constant
  experiment time step : Constant  Value=0.01  SampleTime=0
  enable : Outport
  wires:
  Digital Clock1/1 -> Product/1
  Gain/1 -> Product/2
  Trigonometric Function/1 -> Compare To Zero/1
  Compare To Zero/1 -> Detect Change/1
  Detect Change/1 -> Data Type Conversion/1
  Product/1 -> Add/1
  constant/1 -> Divide/1
  Divide/1 -> Gain/1
  Add/1 -> Trigonometric Function/1
  experiment time step/1 -> Add/2
  Data Type Conversion/1 -> enable/1
  CAM_time_step/1 -> Divide/2

== system V2x_TC_TrafficLights_cs/TrafficSignPoleNL_8/Infrastructure CAM generator/set CAM signals ==
  CAM generation signal : Inport
  warning : Inport  Port=2
  messageID : Inport  Port=3
  actorID : Inport  Port=4
  actorType : Inport  Port=5
  trafficLightPriority : Inport  Port=6
  GPS_lat : Inport  Port=7
  GPS_long : Inport  Port=8
  GPS_alt : Inport  Port=9
  rotZ : Inport  Port=10
  Mux : Mux  Inputs=9
  Switch : Switch  Criteria=u2 > Threshold  Threshold=0.5
  Unit Delay : UnitDelay  SampleTime=-1
  CAM_signals : Outport
  wires:
  rotZ/1 -> Mux/9
  GPS_alt/1 -> Mux/8
  GPS_long/1 -> Mux/7
  GPS_lat/1 -> Mux/6
  trafficLightPriority/1 -> Mux/5
  actorType/1 -> Mux/4
  actorID/1 -> Mux/3
  messageID/1 -> Mux/2
  warning/1 -> Mux/1
  Unit Delay/1 -> Switch/3
  Switch/1 -> CAM_signals/1, Unit Delay/1
  Mux/1 -> Switch/1
  CAM generation signal/1 -> Switch/2

== system V2x_TC_TrafficLights_cs/TrafficSignPoleNL_9/Infrastructure CAM generator/CAM generation ==
  Enable : EnablePort  StatesWhenEnabling=reset  PropagateVarSize=During execution
  Add : Sum  Inputs=+-
  Data Store Memory : DataStoreMemory  DataStoreName=CAM_time
  Data Store Read : DataStoreRead  DataStoreName=CAM_time  SampleTime=0.01
  Digital Clock : DigitalClock  SampleTime=0.01
  Merge : Merge
  if : If  IfExpression=u1 >= 1
  no : Subsystem  TreatAsAtomicUnit=on
  yes : Subsystem  TreatAsAtomicUnit=on
  CAN generation signal : Outport  OutputWhenDisabled=reset  InitialOutput=0
  wires:
  if/2 -> no/Action
  Merge/1 -> CAN generation signal/1
  no/1(Out1) -> Merge/2
  yes/1(Out1) -> Merge/1
  if/1 -> yes/Action
  Add/1 -> if/1
  Data Store Read/1 -> Add/2
  Digital Clock/1 -> Add/1

== system V2x_TC_TrafficLights_cs/TrafficSignPoleNL_9/Infrastructure CAM generator/Enabled Subsystem ==
  CAM_sig : Inport
  Enable : EnablePort  PropagateVarSize=During execution
  CAM_signals : Outport  OutputWhenDisabled=reset  InitialOutput=0
  wires:
  CAM_sig/1 -> CAM_signals/1

== system V2x_TC_TrafficLights_cs/TrafficSignPoleNL_9/Infrastructure CAM generator/coder ==
  R : Inport
  O : Inport  Port=2
  G : Inport  Port=3
  Add : Sum  Inputs=+++
  Gain : Gain  Gain=2
  Gain1 : Gain  Gain=3
  trafficLightPriority : Outport
  wires:
  Gain/1 -> Add/2
  Gain1/1 -> Add/3
  R/1 -> Add/1
  O/1 -> Gain/1
  Add/1 -> trafficLightPriority/1
  G/1 -> Gain1/1

== system V2x_TC_TrafficLights_cs/TrafficSignPoleNL_9/Infrastructure CAM generator/impulse generator ==
  CAM_time_step : Inport
  Add : Sum
  Compare To Zero : simulink/Logic and Bit Operations/Compare To Zero  SystemSampleTime=-1  relop=>  LogicOutDataTypeMode=boolean  ZeroCross=off
  Data Type Conversion : DataTypeConversion  OutDataTypeStr=boolean
  Detect Change : simulink/Logic and Bit Operations/Detect Change  SystemSampleTime=-1  vinit=0
  Digital Clock1 : DigitalClock  SampleTime=0.01
  Divide : Product  Inputs=*/
  Gain : Gain  Gain=pi
  Product : Product  RndMeth=Zero
  Trigonometric Function : Trigonometry
  constant : Constant
  experiment time step : Constant  Value=0.01  SampleTime=0
  enable : Outport
  wires:
  CAM_time_step/1 -> Divide/2
  Data Type Conversion/1 -> enable/1
  experiment time step/1 -> Add/2
  Add/1 -> Trigonometric Function/1
  Divide/1 -> Gain/1
  constant/1 -> Divide/1
  Product/1 -> Add/1
  Detect Change/1 -> Data Type Conversion/1
  Compare To Zero/1 -> Detect Change/1
  Trigonometric Function/1 -> Compare To Zero/1
  Gain/1 -> Product/2
  Digital Clock1/1 -> Product/1

== system V2x_TC_TrafficLights_cs/TrafficSignPoleNL_9/Infrastructure CAM generator/impulse generator1 ==
  CAM_time_step : Inport
  Add : Sum
  Compare To Zero : simulink/Logic and Bit Operations/Compare To Zero  SystemSampleTime=-1  relop=>  LogicOutDataTypeMode=boolean  ZeroCross=off
  Data Type Conversion : DataTypeConversion  OutDataTypeStr=boolean
  Detect Change : simulink/Logic and Bit Operations/Detect Change  SystemSampleTime=-1  vinit=0
  Digital Clock1 : DigitalClock  SampleTime=0.01
  Divide : Product  Inputs=*/
  Gain : Gain  Gain=pi
  Product : Product  RndMeth=Zero
  Trigonometric Function : Trigonometry
  constant : Constant
  experiment time step : Constant  Value=0.01  SampleTime=0
  enable : Outport
  wires:
  Digital Clock1/1 -> Product/1
  Gain/1 -> Product/2
  Trigonometric Function/1 -> Compare To Zero/1
  Compare To Zero/1 -> Detect Change/1
  Detect Change/1 -> Data Type Conversion/1
  Product/1 -> Add/1
  constant/1 -> Divide/1
  Divide/1 -> Gain/1
  Add/1 -> Trigonometric Function/1
  experiment time step/1 -> Add/2
  Data Type Conversion/1 -> enable/1
  CAM_time_step/1 -> Divide/2

== system V2x_TC_TrafficLights_cs/TrafficSignPoleNL_9/Infrastructure CAM generator/set CAM signals ==
  CAM generation signal : Inport
  warning : Inport  Port=2
  messageID : Inport  Port=3
  actorID : Inport  Port=4
  actorType : Inport  Port=5
  trafficLightPriority : Inport  Port=6
  GPS_lat : Inport  Port=7
  GPS_long : Inport  Port=8
  GPS_alt : Inport  Port=9
  rotZ : Inport  Port=10
  Mux : Mux  Inputs=9
  Switch : Switch  Criteria=u2 > Threshold  Threshold=0.5
  Unit Delay : UnitDelay  SampleTime=-1
  CAM_signals : Outport
  wires:
  rotZ/1 -> Mux/9
  GPS_alt/1 -> Mux/8
  GPS_long/1 -> Mux/7
  GPS_lat/1 -> Mux/6
  trafficLightPriority/1 -> Mux/5
  actorType/1 -> Mux/4
  actorID/1 -> Mux/3
  messageID/1 -> Mux/2
  warning/1 -> Mux/1
  Unit Delay/1 -> Switch/3
  Switch/1 -> CAM_signals/1, Unit Delay/1
  Mux/1 -> Switch/1
  CAM generation signal/1 -> Switch/2

== system V2x_TC_TrafficLights_cs/VISUWheels_Audi_A8_1/conv_RotWheelFL/RotMat2AxisAngle ==
  RotMat_Visu : Inport
  Data Type Conversion : DataTypeConversion
  Divide : Product  Inputs=*/
  Gain : Gain  Gain=2
  Mux : Mux  Inputs=3
  Mux1 : Mux  Inputs=2
  Saturation1 : Saturate  UpperLimit=3-0.001  LowerLimit=-1+0.001
  Trigonometric Function : Trigonometry
  U1 : Fcn  Expr=u[6]-u[8]
  U2 : Fcn  Expr=u[7]-u[3]
  U3 : Fcn  Expr=u[2]-u[4]
  U_Phi : Fcn  Expr=u[1]+u[5]+u[9]
  U_Phi1 : Fcn  Expr=acos((u(1)-1)/2)
  U_Phi2 : Fcn  Expr=acos((u(1)-1)/2)
  Angles_Visu : Outport
  wires:
  U_Phi2/1 -> Trigonometric Function/1
  Saturation1/1 -> U_Phi2/1
  U_Phi1/1 -> Data Type Conversion/1
  U_Phi/1 -> Saturation1/1, U_Phi1/1
  Divide/1 -> Mux1/1
  Mux1/1 -> Angles_Visu/1
  Gain/1 -> Divide/2
  Mux/1 -> Divide/1
  U3/1 -> Mux/3
  U2/1 -> Mux/2
  U1/1 -> Mux/1
  Trigonometric Function/1 -> Gain/1
  RotMat_Visu/1 -> U_Phi/1, U3/1, U2/1, U1/1
  Data Type Conversion/1 -> Mux1/2
